simulink model "MX_Gateway"  (Simulink 9.0)
settings: Solver=FixedStepDiscrete  StartTime=0.0  StopTime=100  FixedStep=0.01  RelTol=1e-3
values from neighbouring files (symbols this model uses):
  ACTIVE = Bobcat.Parameter; CreateDefine( ACTIVE                         ,1                          ,'Define' ,'defines.h' )    [defines.m]
  ACTIVE_MODE_ONE = Bobcat.Parameter; CreateDefine( ACTIVE_MODE_ONE                ,19                         ,'Define' ,'defines.h' )    [defines.m]
  AUTHENTICATED = Bobcat.Parameter; CreateDefine( AUTHENTICATED                  ,1                          ,'Define' ,'defines.h' )    [defines.m]
  AUX_EMEA_CONTROLS = Bobcat.Parameter; CreateDefine( AUX_EMEA_CONTROLS  ,1   ,'Define' ,'defines.h' )    [enums.m]
  AUX_FLOW_HIGH = Bobcat.Parameter; CreateDefine( AUX_FLOW_HIGH           ,4  ,'Define' ,'defines.h' )    [enums.m]
  AUX_FLOW_INACTIVE = Bobcat.Parameter; CreateDefine( AUX_FLOW_INACTIVE       ,0  ,'Define' ,'defines.h' )    [enums.m]
  AUX_FLOW_LOW = Bobcat.Parameter; CreateDefine( AUX_FLOW_LOW            ,1  ,'Define' ,'defines.h' )    [enums.m]
  AUX_FLOW_MEDIUM = Bobcat.Parameter; CreateDefine( AUX_FLOW_MEDIUM         ,2  ,'Define' ,'defines.h' )    [enums.m]
  AUX_FLOW_STANDARD = Bobcat.Parameter; CreateDefine( AUX_FLOW_STANDARD       ,3  ,'Define' ,'defines.h' )    [enums.m]
  AUX_NON_FUNCTIONAL = Bobcat.Parameter; CreateDefine( AUX_NON_FUNCTIONAL ,255 ,'Define' ,'defines.h' )    [enums.m]
  BASE_ACTIVE = Bobcat.Parameter; CreateDefine( BASE_ACTIVE                    ,253                        ,'Define' ,'defines.h' )    [defines.m]
  BASE_DETENT = Bobcat.Parameter; CreateDefine( BASE_DETENT                    ,1                          ,'Define' ,'defines.h' )    [defines.m]
  BEEP_NONE = Bobcat.Parameter; CreateDefine( BEEP_NONE                      ,255                        ,'Define' ,'defines.h' )    [defines.m]
  CD_AIR_FILTER_DEBOUNCE_NORMAL = Bobcat.Parameter; CreateAliasCD( CD_AIR_FILTER_DEBOUNCE_NORMAL                        ,uint16(500)                ,'ConstantDataPointer' ,'CONSTANT_DATA' ,'AIR_FILTER_DEBOUNCE_NORMAL')    [cdata.m]    (also in hc12_cdata.m; m4_cdata.m)
  CD_AIR_FILTER_DEBOUNCE_NOT_CONNECTED = Bobcat.Parameter; CreateAliasCD( CD_AIR_FILTER_DEBOUNCE_NOT_CONNECTED                 ,uint16(3000)               ,'ConstantDataPointer' ,'CONSTANT_DATA' ,'AIR_FILTER_DEBOUNCE_NOT_CONNECTED')    [cdata.m]    (also in hc12_cdata.m; m4_cdata.m)
  CD_AIR_FILTER_DEBOUNCE_PLUGGED = Bobcat.Parameter; CreateAliasCD( CD_AIR_FILTER_DEBOUNCE_PLUGGED                       ,uint16(3000)               ,'ConstantDataPointer' ,'CONSTANT_DATA' ,'AIR_FILTER_DEBOUNCE_PLUGGED')    [cdata.m]    (also in hc12_cdata.m; m4_cdata.m)
  CD_AIR_FILTER_FUNCTION = Bobcat.Parameter; CreateAliasCD( CD_AIR_FILTER_FUNCTION                               ,uint8(255)                 ,'ConstantDataPointer' ,'CONSTANT_DATA' ,'AIR_FILTER_FUNCTION')    [cdata.m]    (also in hc12_cdata.m; m4_cdata.m)
  CD_AIR_FILTER_PLUGGED_WEIGHT = Bobcat.Parameter; CreateAliasCD( CD_AIR_FILTER_PLUGGED_WEIGHT                         ,uint8(2)                   ,'ConstantDataPointer' ,'CONSTANT_DATA' ,'AIR_FILTER_PLUGGED_WEIGHT')    [cdata.m]    (also in hc12_cdata.m; m4_cdata.m)
  CD_AIR_FILTER_UNPLUGGED_WEIGHT = Bobcat.Parameter; CreateAliasCD( CD_AIR_FILTER_UNPLUGGED_WEIGHT                       ,uint8(1)                   ,'ConstantDataPointer' ,'CONSTANT_DATA' ,'AIR_FILTER_UNPLUGGED_WEIGHT')    [cdata.m]    (also in hc12_cdata.m; m4_cdata.m)
  CD_AUTOIDLE_ARMBAR_DELAY = Bobcat.Parameter; CreateAliasCD( CD_AUTOIDLE_ARMBAR_DELAY                             ,uint8(2)                   ,'ConstantDataPointer' ,'CONSTANT_DATA' ,'AUTOIDLE_ARMBAR_DELAY')    [cdata.m]    (also in hc12_cdata.m; m4_cdata.m)
  CD_AUTOIDLE_FUNCTION = Bobcat.Parameter; CreateAliasCD( CD_AUTOIDLE_FUNCTION                                 ,uint8(255)                 ,'ConstantDataPointer' ,'CONSTANT_DATA' ,'AUTOIDLE_FUNCTION')    [cdata.m]    (also in hc12_cdata.m; m4_cdata.m)
  CD_AUTOIDLE_IDLE_DELAY = Bobcat.Parameter; CreateAliasCD( CD_AUTOIDLE_IDLE_DELAY                               ,uint8(40)                  ,'ConstantDataPointer' ,'CONSTANT_DATA' ,'AUTOIDLE_IDLE_DELAY')    [cdata.m]    (also in hc12_cdata.m; m4_cdata.m)
  CD_AUTOIDLE_LOW_IDLE_START = Bobcat.Parameter; CreateAliasCD( CD_AUTOIDLE_LOW_IDLE_START                           ,uint8(255)                 ,'ConstantDataPointer' ,'CONSTANT_DATA' ,'AUTOIDLE_LOW_IDLE_START')    [cdata.m]    (also in hc12_cdata.m; m4_cdata.m)
  CD_AUTOIDLE_PRESSURE_OFF = Bobcat.Parameter; CreateAliasCD( CD_AUTOIDLE_PRESSURE_OFF                             ,uint16(300)                ,'ConstantDataPointer' ,'CONSTANT_DATA' ,'AUTOIDLE_PRESSURE_OFF')    [cdata.m]    (also in hc12_cdata.m; m4_cdata.m)
  CD_AUTOIDLE_PRESSURE_ON = Bobcat.Parameter; CreateAliasCD( CD_AUTOIDLE_PRESSURE_ON                              ,uint16(270)                ,'ConstantDataPointer' ,'CONSTANT_DATA' ,'AUTOIDLE_PRESSURE_ON')    [cdata.m]    (also in hc12_cdata.m; m4_cdata.m)
  CD_AUTOSHIFT_FUNCTION = Bobcat.Parameter; CreateAliasCD( CD_AUTOSHIFT_FUNCTION                                ,uint8(255)                 ,'ConstantDataPointer' ,'CONSTANT_DATA' ,'AUTOSHIFT_FUNCTION')    [cdata.m]    (also in hc12_cdata.m; m4_cdata.m)
  CD_AUTOSHIFT_PHIGH1 = Bobcat.Parameter; CreateAliasCD( CD_AUTOSHIFT_PHIGH1                                  ,uint16(205)                ,'ConstantDataPointer' ,'CONSTANT_DATA' ,'AUTOSHIFT_PHIGH1')    [cdata.m]    (also in hc12_cdata.m; m4_cdata.m)
  CD_AUTOSHIFT_PHIGH2 = Bobcat.Parameter; CreateAliasCD( CD_AUTOSHIFT_PHIGH2                                  ,uint16(193)                ,'ConstantDataPointer' ,'CONSTANT_DATA' ,'AUTOSHIFT_PHIGH2')    [cdata.m]    (also in hc12_cdata.m; m4_cdata.m)
  CD_AUTOSHIFT_PHIGH_TEMP1 = Bobcat.Parameter; CreateAliasCD( CD_AUTOSHIFT_PHIGH_TEMP1                             ,int16(70)                  ,'ConstantDataPointer' ,'CONSTANT_DATA' ,'AUTOSHIFT_PHIGH_TEMP1')    [cdata.m]    (also in hc12_cdata.m; m4_cdata.m)
  CD_AUTOSHIFT_PHIGH_TEMP2 = Bobcat.Parameter; CreateAliasCD( CD_AUTOSHIFT_PHIGH_TEMP2                             ,int16(160)                 ,'ConstantDataPointer' ,'CONSTANT_DATA' ,'AUTOSHIFT_PHIGH_TEMP2')    [cdata.m]    (also in hc12_cdata.m; m4_cdata.m)
  CD_AUTOSHIFT_PLOW1 = Bobcat.Parameter; CreateAliasCD( CD_AUTOSHIFT_PLOW1                                   ,uint16(130)                ,'ConstantDataPointer' ,'CONSTANT_DATA' ,'AUTOSHIFT_PLOW1')    [cdata.m]    (also in hc12_cdata.m; m4_cdata.m)
  CD_AUTOSHIFT_PLOW2 = Bobcat.Parameter; CreateAliasCD( CD_AUTOSHIFT_PLOW2                                   ,uint16(100)                ,'ConstantDataPointer' ,'CONSTANT_DATA' ,'AUTOSHIFT_PLOW2')    [cdata.m]    (also in hc12_cdata.m; m4_cdata.m)
  CD_AUTOSHIFT_PLOW_TEMP1 = Bobcat.Parameter; CreateAliasCD( CD_AUTOSHIFT_PLOW_TEMP1                              ,int16(20)                  ,'ConstantDataPointer' ,'CONSTANT_DATA' ,'AUTOSHIFT_PLOW_TEMP1')    [cdata.m]    (also in hc12_cdata.m; m4_cdata.m)
  CD_AUTOSHIFT_PLOW_TEMP2 = Bobcat.Parameter; CreateAliasCD( CD_AUTOSHIFT_PLOW_TEMP2                              ,int16(80)                  ,'ConstantDataPointer' ,'CONSTANT_DATA' ,'AUTOSHIFT_PLOW_TEMP2')    [cdata.m]    (also in hc12_cdata.m; m4_cdata.m)
  CD_AUTOSHIFT_TRAVEL_DELAY = Bobcat.Parameter; CreateAliasCD( CD_AUTOSHIFT_TRAVEL_DELAY                            ,uint8(32)                  ,'ConstantDataPointer' ,'CONSTANT_DATA' ,'AUTOSHIFT_TRAVEL_DELAY')    [cdata.m]    (also in hc12_cdata.m; m4_cdata.m)
  CD_AUX2_CRACKPOINT_CURRENT = Bobcat.Parameter; CreateAliasCD( CD_AUX2_CRACKPOINT_CURRENT                           ,fi(0,0,16,12,'hex','0c28') ,'ConstantDataPointer' ,'CONSTANT_DATA' ,'AUX2_CRACKPOINT_CURRENT')    [cdata.m]    (also in hc12_cdata.m; m4_cdata.m)
  CD_AUX2_FLOW_LOW_FUNCTION = Bobcat.Parameter; CreateAliasCD( CD_AUX2_FLOW_LOW_FUNCTION                            ,uint8(255)                 ,'ConstantDataPointer' ,'CONSTANT_DATA' ,'AUX2_FLOW_LOW_FUNCTION')    [cdata.m]    (also in hc12_cdata.m; m4_cdata.m)
  CD_AUX2_FLOW_MED_FUNCTION = Bobcat.Parameter; CreateAliasCD( CD_AUX2_FLOW_MED_FUNCTION                            ,uint8(255)                 ,'ConstantDataPointer' ,'CONSTANT_DATA' ,'AUX2_FLOW_MED_FUNCTION')    [cdata.m]    (also in hc12_cdata.m; m4_cdata.m)
  CD_AUX2_HI_FLOW_PERCENT = Bobcat.Parameter; CreateAliasCD( CD_AUX2_HI_FLOW_PERCENT                              ,fi(0,0,16,16,'hex','ffff') ,'ConstantDataPointer' ,'CONSTANT_DATA' ,'AUX2_HI_FLOW_PERCENT')    [cdata.m]    (also in hc12_cdata.m; m4_cdata.m)
  CD_AUX2_LOW_FLOW_PERCENT = Bobcat.Parameter; CreateAliasCD( CD_AUX2_LOW_FLOW_PERCENT                             ,fi(0,0,16,16,'hex','7333') ,'ConstantDataPointer' ,'CONSTANT_DATA' ,'AUX2_LOW_FLOW_PERCENT')    [cdata.m]    (also in hc12_cdata.m; m4_cdata.m)
  CD_AUX2_MAX_FLOW_CURRENT = Bobcat.Parameter; CreateAliasCD( CD_AUX2_MAX_FLOW_CURRENT                             ,fi(0,0,16,12,'hex','14cc') ,'ConstantDataPointer' ,'CONSTANT_DATA' ,'AUX2_MAX_FLOW_CURRENT')    [cdata.m]    (also in hc12_cdata.m; m4_cdata.m)
  CD_AUX2_MED_FLOW_PERCENT = Bobcat.Parameter; CreateAliasCD( CD_AUX2_MED_FLOW_PERCENT                             ,fi(0,0,16,16,'hex','9999') ,'ConstantDataPointer' ,'CONSTANT_DATA' ,'AUX2_MED_FLOW_PERCENT')    [cdata.m]    (also in hc12_cdata.m; m4_cdata.m)
  CD_AUX_CRACKPOINT_CURRENT = Bobcat.Parameter; CreateAliasCD( CD_AUX_CRACKPOINT_CURRENT                            ,fi(0,0,16,12,'hex','0000') ,'ConstantDataPointer' ,'CONSTANT_DATA' ,'AUX_CRACKPOINT_CURRENT')    [cdata.m]    (also in hc12_cdata.m; m4_cdata.m)
  CD_AUX_DETENT_FLASH_TIME = Bobcat.Parameter; CreateAliasCD( CD_AUX_DETENT_FLASH_TIME                             ,uint16(72)                 ,'ConstantDataPointer' ,'CONSTANT_DATA' ,'AUX_DETENT_FLASH_TIME')    [cdata.m]    (also in hc12_cdata.m; m4_cdata.m)
  CD_AUX_DETENT_HOLD_TIME = Bobcat.Parameter; CreateAliasCD( CD_AUX_DETENT_HOLD_TIME                              ,uint16(100)                ,'ConstantDataPointer' ,'CONSTANT_DATA' ,'AUX_DETENT_HOLD_TIME')    [cdata.m]    (also in hc12_cdata.m; m4_cdata.m)
  CD_AUX_FULL_ON_AUX1 = Bobcat.Parameter; CreateAliasCD( CD_AUX_FULL_ON_AUX1                                  ,fi(0,0,16,12,'hex','10cd') ,'ConstantDataPointer' ,'CONSTANT_DATA' ,'AUX_FULL_ON_AUX1')    [cdata.m]    (also in hc12_cdata.m; m4_cdata.m)
  CD_AUX_FULL_ON_AUX2 = Bobcat.Parameter; CreateAliasCD( CD_AUX_FULL_ON_AUX2                                  ,fi(0,0,16,12,'hex','139d') ,'ConstantDataPointer' ,'CONSTANT_DATA' ,'AUX_FULL_ON_AUX2')    [cdata.m]    (also in hc12_cdata.m; m4_cdata.m)
  CD_AUX_FULL_ON_AUX3 = Bobcat.Parameter; CreateAliasCD( CD_AUX_FULL_ON_AUX3                                  ,fi(0,0,16,12,'hex','17d7') ,'ConstantDataPointer' ,'CONSTANT_DATA' ,'AUX_FULL_ON_AUX3')    [cdata.m]    (also in hc12_cdata.m; m4_cdata.m)
  CD_AUX_FUNCTION = Bobcat.Parameter; CreateAliasCD( CD_AUX_FUNCTION                                      ,uint8(0)                   ,'ConstantDataPointer' ,'CONSTANT_DATA' ,'AUX_FUNCTION')    [cdata.m]    (also in hc12_cdata.m; m4_cdata.m)
  CD_AUX_RATE_LIMIT_CRACKPOINT = Bobcat.Parameter; CreateAliasCD( CD_AUX_RATE_LIMIT_CRACKPOINT                         ,fi(0,0,16,12,'hex','0800') ,'ConstantDataPointer' ,'CONSTANT_DATA' ,'AUX_RATE_LIMIT_CRACKPOINT')    [cdata.m]    (also in hc12_cdata.m; m4_cdata.m)
  CD_AUX_RATE_LIMIT_CRACKPOINT_RATE = Bobcat.Parameter; CreateAliasCD( CD_AUX_RATE_LIMIT_CRACKPOINT_RATE                    ,fi(0,1,32,12,'int',102400) ,'ConstantDataPointer' ,'CONSTANT_DATA' ,'AUX_RATE_LIMIT_CRACKPOINT_RATE')    [cdata.m]    (also in hc12_cdata.m; m4_cdata.m)
  CD_AUX_RATE_LIMIT_FUNCTION = Bobcat.Parameter; CreateAliasCD( CD_AUX_RATE_LIMIT_FUNCTION                           ,uint8(255)                 ,'ConstantDataPointer' ,'CONSTANT_DATA' ,'AUX_RATE_LIMIT_FUNCTION')    [cdata.m]    (also in hc12_cdata.m; m4_cdata.m)
  CD_AUX_RATE_LIMIT_MAX_ENG_SPD = Bobcat.Parameter; CreateAliasCD( CD_AUX_RATE_LIMIT_MAX_ENG_SPD                        ,uint16(1600)               ,'ConstantDataPointer' ,'CONSTANT_DATA' ,'AUX_RATE_LIMIT_MAX_ENG_SPD')    [cdata.m]    (also in hc12_cdata.m; m4_cdata.m)
  CD_AUX_RATE_LIMIT_MIN_ENG_SPD = Bobcat.Parameter; CreateAliasCD( CD_AUX_RATE_LIMIT_MIN_ENG_SPD                        ,uint16(100)                ,'ConstantDataPointer' ,'CONSTANT_DATA' ,'AUX_RATE_LIMIT_MIN_ENG_SPD')    [cdata.m]    (also in hc12_cdata.m; m4_cdata.m)
  CD_AUX_RATE_LIMIT_NORMAL_RATE = Bobcat.Parameter; CreateAliasCD( CD_AUX_RATE_LIMIT_NORMAL_RATE                        ,fi(0,1,32,12,'int',2048)   ,'ConstantDataPointer' ,'CONSTANT_DATA' ,'AUX_RATE_LIMIT_NORMAL_RATE')    [cdata.m]    (also in hc12_cdata.m; m4_cdata.m)
  CD_AUXPOT_CAL_DEADBAND_HIGH = Bobcat.Parameter; CreateAliasCD( CD_AUXPOT_CAL_DEADBAND_HIGH                          ,uint16(574)                ,'ConstantDataPointer' ,'CONSTANT_DATA' ,'AUXPOT_CAL_DEADBAND_HIGH')    [cdata.m]    (also in hc12_cdata.m; m4_cdata.m)
  CD_AUXPOT_CAL_DEADBAND_LOW = Bobcat.Parameter; CreateAliasCD( CD_AUXPOT_CAL_DEADBAND_LOW                           ,uint16(424)                ,'ConstantDataPointer' ,'CONSTANT_DATA' ,'AUXPOT_CAL_DEADBAND_LOW')    [cdata.m]    (also in hc12_cdata.m; m4_cdata.m)
  CD_AUXPOT_CENTER_RATIO = Bobcat.Parameter; CreateAliasCD( CD_AUXPOT_CENTER_RATIO                               ,uint16(500)                ,'ConstantDataPointer' ,'CONSTANT_DATA' ,'AUXPOT_CENTER_RATIO')    [cdata.m]    (also in hc12_cdata.m; m4_cdata.m)
  CD_AUXPOT_DEADBAND = Bobcat.Parameter; CreateAliasCD( CD_AUXPOT_DEADBAND                                   ,uint8(38)                  ,'ConstantDataPointer' ,'CONSTANT_DATA' ,'AUXPOT_DEADBAND')    [cdata.m]    (also in hc12_cdata.m; m4_cdata.m)
  CD_AUXPOT_FUNCTION = Bobcat.Parameter; CreateAliasCD( CD_AUXPOT_FUNCTION                                   ,uint8(0)                   ,'ConstantDataPointer' ,'CONSTANT_DATA' ,'AUXPOT_FUNCTION')    [cdata.m]    (also in hc12_cdata.m; m4_cdata.m)
  CD_AUXPOT_RATIO_GAIN = Bobcat.Parameter; CreateAliasCD( CD_AUXPOT_RATIO_GAIN                                 ,uint16(1000)               ,'ConstantDataPointer' ,'CONSTANT_DATA' ,'AUXPOT_RATIO_GAIN')    [cdata.m]    (also in hc12_cdata.m; m4_cdata.m)
  CD_CAN_THROTTLE_FUNCTION = Bobcat.Parameter; CreateAliasCD( CD_CAN_THROTTLE_FUNCTION                             ,uint8(255)                 ,'ConstantDataPointer' ,'CONSTANT_DATA' ,'CAN_THROTTLE_FUNCTION')    [cdata.m]    (also in hc12_cdata.m; m4_cdata.m)
  CD_CAN_THROTTLE_REVERSE = Bobcat.Parameter; CreateAliasCD( CD_CAN_THROTTLE_REVERSE                              ,uint8(255)                 ,'ConstantDataPointer' ,'CONSTANT_DATA' ,'CAN_THROTTLE_REVERSE')    [cdata.m]    (also in hc12_cdata.m; m4_cdata.m)
  CD_CONSOLE_DEBOUNCE_ABSENT = Bobcat.Parameter; CreateAliasCD( CD_CONSOLE_DEBOUNCE_ABSENT                           ,uint16(12)                 ,'ConstantDataPointer' ,'CONSTANT_DATA' ,'CONSOLE_DEBOUNCE_ABSENT')    [cdata.m]    (also in hc12_cdata.m; m4_cdata.m)
  CD_CONSOLE_DEBOUNCE_OORH = Bobcat.Parameter; CreateAliasCD( CD_CONSOLE_DEBOUNCE_OORH                             ,uint16(12)                 ,'ConstantDataPointer' ,'CONSTANT_DATA' ,'CONSOLE_DEBOUNCE_OORH')    [cdata.m]    (also in hc12_cdata.m; m4_cdata.m)
  CD_CONSOLE_DEBOUNCE_OORL = Bobcat.Parameter; CreateAliasCD( CD_CONSOLE_DEBOUNCE_OORL                             ,uint16(12)                 ,'ConstantDataPointer' ,'CONSTANT_DATA' ,'CONSOLE_DEBOUNCE_OORL')    [cdata.m]    (also in hc12_cdata.m; m4_cdata.m)
  CD_CONSOLE_DEBOUNCE_PRESENT = Bobcat.Parameter; CreateAliasCD( CD_CONSOLE_DEBOUNCE_PRESENT                          ,uint16(12)                 ,'ConstantDataPointer' ,'CONSTANT_DATA' ,'CONSOLE_DEBOUNCE_PRESENT')    [cdata.m]    (also in hc12_cdata.m; m4_cdata.m)
  CD_CONSOLE_FUNCTION = Bobcat.Parameter; CreateAliasCD( CD_CONSOLE_FUNCTION                                  ,uint8(0)                   ,'ConstantDataPointer' ,'CONSTANT_DATA' ,'CONSOLE_FUNCTION')    [cdata.m]    (also in hc12_cdata.m; m4_cdata.m)
  CD_CONSOLE_OORH = Bobcat.Parameter; CreateAliasCD( CD_CONSOLE_OORH                                      ,uint16(740)                ,'ConstantDataPointer' ,'CONSTANT_DATA' ,'CONSOLE_OORH')    [cdata.m]    (also in hc12_cdata.m; m4_cdata.m)
  CD_CONSOLE_OORL = Bobcat.Parameter; CreateAliasCD( CD_CONSOLE_OORL                                      ,uint16(10)                 ,'ConstantDataPointer' ,'CONSTANT_DATA' ,'CONSOLE_OORL')    [cdata.m]    (also in hc12_cdata.m; m4_cdata.m)
  CD_CONSOLE_PRESENT_HIGH = Bobcat.Parameter; CreateAliasCD( CD_CONSOLE_PRESENT_HIGH                              ,uint16(515)                ,'ConstantDataPointer' ,'CONSTANT_DATA' ,'CONSOLE_PRESENT_HIGH')    [cdata.m]    (also in hc12_cdata.m; m4_cdata.m)
  CD_CONSOLE_PRESENT_LOW = Bobcat.Parameter; CreateAliasCD( CD_CONSOLE_PRESENT_LOW                               ,uint16(375)                ,'ConstantDataPointer' ,'CONSTANT_DATA' ,'CONSOLE_PRESENT_LOW')    [cdata.m]    (also in hc12_cdata.m; m4_cdata.m)
  CD_DELUXE_GEN5_5_FUNCTION = Bobcat.Parameter; CreateAliasCD( CD_DELUXE_GEN5_5_FUNCTION                            ,uint8(255)                 ,'ConstantDataPointer' ,'CONSTANT_DATA' ,'DELUXE_GEN5_5_FUNCTION')    [cdata.m]    (also in hc12_cdata.m; m4_cdata.m)
  CD_DELUXE_GEN5_FUNCTION = Bobcat.Parameter; CreateAliasCD( CD_DELUXE_GEN5_FUNCTION                              ,uint8(255)                 ,'ConstantDataPointer' ,'CONSTANT_DATA' ,'DELUXE_GEN5_FUNCTION')    [cdata.m]    (also in hc12_cdata.m; m4_cdata.m)
  CD_DERATE_ENGINE_SPEED_DEBOUNCE_HIGH = Bobcat.Parameter; CreateAliasCD( CD_DERATE_ENGINE_SPEED_DEBOUNCE_HIGH                 ,uint16(200)                ,'ConstantDataPointer' ,'CONSTANT_DATA' ,'DERATE_ENGINE_SPEED_DEBOUNCE_HIGH')    [cdata.m]    (also in hc12_cdata.m; m4_cdata.m)
  CD_DERATE_ENGINE_SPEED_DEBOUNCE_SHUTDOWN = Bobcat.Parameter; CreateAliasCD( CD_DERATE_ENGINE_SPEED_DEBOUNCE_SHUTDOWN             ,uint16(1000)               ,'ConstantDataPointer' ,'CONSTANT_DATA' ,'DERATE_ENGINE_SPEED_DEBOUNCE_SHUTDOWN')    [cdata.m]    (also in hc12_cdata.m; m4_cdata.m)
  CD_DERATE_ENGINE_SPEED_HIGH_OFFSET = Bobcat.Parameter; CreateAliasCD( CD_DERATE_ENGINE_SPEED_HIGH_OFFSET                   ,uint16(200)                ,'ConstantDataPointer' ,'CONSTANT_DATA' ,'DERATE_ENGINE_SPEED_HIGH_OFFSET')    [cdata.m]    (also in hc12_cdata.m; m4_cdata.m)
  CD_DERATE_METHOD = Bobcat.Parameter; CreateAliasCD( CD_DERATE_METHOD                                     ,uint8(0)                   ,'ConstantDataPointer' ,'CONSTANT_DATA' ,'DERATE_METHOD')    [cdata.m]    (also in hc12_cdata.m; m4_cdata.m)
  CD_DUMP_VALVE_FUNCTION = Bobcat.Parameter; CreateAliasCD( CD_DUMP_VALVE_FUNCTION                               ,uint8(255)                 ,'ConstantDataPointer' ,'CONSTANT_DATA' ,'DUMP_VALVE_FUNCTION')    [cdata.m]    (also in hc12_cdata.m; m4_cdata.m)
  CD_DUMP_VALVE_ON_CURRENT = Bobcat.Parameter; CreateAliasCD( CD_DUMP_VALVE_ON_CURRENT                             ,fi(0,0,16,12,'hex','ffff') ,'ConstantDataPointer' ,'CONSTANT_DATA' ,'DUMP_VALVE_ON_CURRENT')    [cdata.m]    (also in hc12_cdata.m; m4_cdata.m)
  CD_ECO_MODE_THROTTLE_REDUCTION = Bobcat.Parameter; CreateAliasCD( CD_ECO_MODE_THROTTLE_REDUCTION                       ,uint16(850)                ,'ConstantDataPointer' ,'CONSTANT_DATA' ,'ECO_MODE_THROTTLE_REDUCTION')    [cdata.m]    (also in hc12_cdata.m; m4_cdata.m)
  CD_ECT_FILTER_GAIN = Bobcat.Parameter; CreateAliasCD( CD_ECT_FILTER_GAIN                                   ,uint8(6)                   ,'ConstantDataPointer' ,'CONSTANT_DATA' ,'ECT_FILTER_GAIN')    [cdata.m]    (also in hc12_cdata.m; m4_cdata.m)
  CD_ECU_FUNCTION = Bobcat.Parameter; CreateAliasCD( CD_ECU_FUNCTION                                      ,uint8(255)                 ,'ConstantDataPointer' ,'CONSTANT_DATA' ,'ECU_FUNCTION')    [cdata.m]    (also in hc12_cdata.m; m4_cdata.m)
  CD_ECU_HOLD_DEBOUNCE_ERROR_ON = Bobcat.Parameter; CreateAliasCD( CD_ECU_HOLD_DEBOUNCE_ERROR_ON                        ,uint16(20)                 ,'ConstantDataPointer' ,'CONSTANT_DATA' ,'ECU_HOLD_DEBOUNCE_ERROR_ON')    [cdata.m]    (also in hc12_cdata.m; m4_cdata.m)
  CD_EMEA_CONTROLS_HOLD_TIME = Bobcat.Parameter; CreateAliasCD( CD_EMEA_CONTROLS_HOLD_TIME                           ,uint16(30)                 ,'ConstantDataPointer' ,'CONSTANT_DATA' ,'EMEA_CONTROLS_HOLD_TIME')    [cdata.m]    (also in hc12_cdata.m; m4_cdata.m)
  CD_EMEA_CONTROLS_OPTION_A = Bobcat.Parameter; CreateAliasCD( CD_EMEA_CONTROLS_OPTION_A                            ,uint8(255)                 ,'ConstantDataPointer' ,'CONSTANT_DATA' ,'EMEA_CONTROLS_OPTION_A')    [cdata.m]    (also in hc12_cdata.m; m4_cdata.m)
  CD_EMEA_CONTROLS_OPTION_C = Bobcat.Parameter; CreateAliasCD( CD_EMEA_CONTROLS_OPTION_C                            ,uint8(255)                 ,'ConstantDataPointer' ,'CONSTANT_DATA' ,'EMEA_CONTROLS_OPTION_C')    [cdata.m]    (also in hc12_cdata.m; m4_cdata.m)
  CD_EMEA_CONTROLS_REVERSE = Bobcat.Parameter; CreateAliasCD( CD_EMEA_CONTROLS_REVERSE                             ,uint8(255)                 ,'ConstantDataPointer' ,'CONSTANT_DATA' ,'EMEA_CONTROLS_REVERSE')    [cdata.m]    (also in hc12_cdata.m; m4_cdata.m)
  CD_EMEA_ELECTRIC_VERSION = Bobcat.Parameter; CreateAliasCD( CD_EMEA_ELECTRIC_VERSION                             ,uint8(0)                   ,'ConstantDataPointer' ,'CONSTANT_DATA' ,'EMEA_ELECTRIC_VERSION')    [cdata.m]    (also in hc12_cdata.m; m4_cdata.m)
  CD_EMEA_POT_CAL_DEADBAND_HIGH = Bobcat.Parameter; CreateAliasCD( CD_EMEA_POT_CAL_DEADBAND_HIGH                        ,uint16(574)                ,'ConstantDataPointer' ,'CONSTANT_DATA' ,'EMEA_POT_CAL_DEADBAND_HIGH')    [cdata.m]    (also in hc12_cdata.m; m4_cdata.m)
  CD_EMEA_POT_CAL_DEADBAND_LOW = Bobcat.Parameter; CreateAliasCD( CD_EMEA_POT_CAL_DEADBAND_LOW                         ,uint16(424)                ,'ConstantDataPointer' ,'CONSTANT_DATA' ,'EMEA_POT_CAL_DEADBAND_LOW')    [cdata.m]    (also in hc12_cdata.m; m4_cdata.m)
  CD_ENGINE_RPM_FACTOR = Bobcat.Parameter; CreateAliasCD( CD_ENGINE_RPM_FACTOR                                 ,uint8(35)                  ,'ConstantDataPointer' ,'CONSTANT_DATA' ,'ENGINE_RPM_FACTOR')    [cdata.m]    (also in hc12_cdata.m; m4_cdata.m)
  CD_ENGINE_SPEED_FILTER_FAST_VALUE = Bobcat.Parameter; CreateAliasCD( CD_ENGINE_SPEED_FILTER_FAST_VALUE                    ,uint8(1)                   ,'ConstantDataPointer' ,'CONSTANT_DATA' ,'ENGINE_SPEED_FILTER_FAST_VALUE')    [cdata.m]    (also in hc12_cdata.m; m4_cdata.m)
  CD_ENGINE_SPEED_FILTER_SLOW_VALUE = Bobcat.Parameter; CreateAliasCD( CD_ENGINE_SPEED_FILTER_SLOW_VALUE                    ,uint8(4)                   ,'ConstantDataPointer' ,'CONSTANT_DATA' ,'ENGINE_SPEED_FILTER_SLOW_VALUE')    [cdata.m]    (also in hc12_cdata.m; m4_cdata.m)
  CD_ENGINE_SPEED_RUNNING = Bobcat.Parameter; CreateAliasCD( CD_ENGINE_SPEED_RUNNING                              ,uint16(800)                ,'ConstantDataPointer' ,'CONSTANT_DATA' ,'ENGINE_SPEED_RUNNING')    [cdata.m]    (also in hc12_cdata.m; m4_cdata.m)
  CD_ENGINE_START_DELAY_DEBOUNCE_RPM_NO_SIGNAL = Bobcat.Parameter; CreateAliasCD( CD_ENGINE_START_DELAY_DEBOUNCE_RPM_NO_SIGNAL         ,uint16(0)                  ,'ConstantDataPointer' ,'CONSTANT_DATA' ,'ENGINE_START_DELAY_DEBOUNCE_RPM_NO_SIGNAL')    [cdata.m]    (also in hc12_cdata.m; m4_cdata.m)
  CD_ENGINE_START_DELAY_OFF_TIME = Bobcat.Parameter; CreateAliasCD( CD_ENGINE_START_DELAY_OFF_TIME                       ,uint16(0)                  ,'ConstantDataPointer' ,'CONSTANT_DATA' ,'ENGINE_START_DELAY_OFF_TIME')    [cdata.m]    (also in hc12_cdata.m; m4_cdata.m)
  CD_ENGINE_START_DELAY_RPM_NO_SIGNAL = Bobcat.Parameter; CreateAliasCD( CD_ENGINE_START_DELAY_RPM_NO_SIGNAL                  ,uint16(0)                  ,'ConstantDataPointer' ,'CONSTANT_DATA' ,'ENGINE_START_DELAY_RPM_NO_SIGNAL')    [cdata.m]    (also in hc12_cdata.m; m4_cdata.m)
  CD_EOP_DEBOUNCE_EXTREMELY_LOW = Bobcat.Parameter; CreateAliasCD( CD_EOP_DEBOUNCE_EXTREMELY_LOW                        ,uint16(50)                 ,'ConstantDataPointer' ,'CONSTANT_DATA' ,'EOP_DEBOUNCE_EXTREMELY_LOW')    [cdata.m]    (also in hc12_cdata.m; m4_cdata.m)
  CD_EOP_DEBOUNCE_NORMAL = Bobcat.Parameter; CreateAliasCD( CD_EOP_DEBOUNCE_NORMAL                               ,uint16(50)                 ,'ConstantDataPointer' ,'CONSTANT_DATA' ,'EOP_DEBOUNCE_NORMAL')    [cdata.m]    (also in hc12_cdata.m; m4_cdata.m)
  CD_EOP_DEBOUNCE_SHUTDOWN = Bobcat.Parameter; CreateAliasCD( CD_EOP_DEBOUNCE_SHUTDOWN                             ,uint16(100)                ,'ConstantDataPointer' ,'CONSTANT_DATA' ,'EOP_DEBOUNCE_SHUTDOWN')    [cdata.m]    (also in hc12_cdata.m; m4_cdata.m)
  CD_EOP_FILTER_VALUE = Bobcat.Parameter; CreateAliasCD( CD_EOP_FILTER_VALUE                                  ,uint8(3)                   ,'ConstantDataPointer' ,'CONSTANT_DATA' ,'EOP_FILTER_VALUE')    [cdata.m]    (also in hc12_cdata.m; m4_cdata.m)
  CD_EOP_FUNCTION = Bobcat.Parameter; CreateAliasCD( CD_EOP_FUNCTION                                      ,uint8(1)                   ,'ConstantDataPointer' ,'CONSTANT_DATA' ,'EOP_FUNCTION')    [cdata.m]    (also in hc12_cdata.m; m4_cdata.m)
  CD_EOP_SWITCH_GAUGE_VALUE = Bobcat.Parameter; CreateAliasCD( CD_EOP_SWITCH_GAUGE_VALUE                            ,uint16(125)                ,'ConstantDataPointer' ,'CONSTANT_DATA' ,'EOP_SWITCH_GAUGE_VALUE')    [cdata.m]    (also in hc12_cdata.m; m4_cdata.m)
  CD_EOP_SWITCH_LOW = Bobcat.Parameter; CreateAliasCD( CD_EOP_SWITCH_LOW                                    ,uint16(50)                 ,'ConstantDataPointer' ,'CONSTANT_DATA' ,'EOP_SWITCH_LOW')    [cdata.m]    (also in hc12_cdata.m; m4_cdata.m)
  CD_EOP_SWITCH_PRESSURE_VALUE = Bobcat.Parameter; CreateAliasCD( CD_EOP_SWITCH_PRESSURE_VALUE                         ,uint16(40)                 ,'ConstantDataPointer' ,'CONSTANT_DATA' ,'EOP_SWITCH_PRESSURE_VALUE')    [cdata.m]    (also in hc12_cdata.m; m4_cdata.m)
  CD_EOT_DEBOUNCE_EXTREMELY_LOW = Bobcat.Parameter; CreateAliasCD( CD_EOT_DEBOUNCE_EXTREMELY_LOW                        ,uint16(2)                  ,'ConstantDataPointer' ,'CONSTANT_DATA' ,'EOT_DEBOUNCE_EXTREMELY_LOW')    [cdata.m]    (also in hc12_cdata.m; m4_cdata.m)
  CD_EOT_DEBOUNCE_LOW = Bobcat.Parameter; CreateAliasCD( CD_EOT_DEBOUNCE_LOW                                  ,uint16(2)                  ,'ConstantDataPointer' ,'CONSTANT_DATA' ,'EOT_DEBOUNCE_LOW')    [cdata.m]    (also in hc12_cdata.m; m4_cdata.m)
  CD_EOT_DEBOUNCE_NORMAL = Bobcat.Parameter; CreateAliasCD( CD_EOT_DEBOUNCE_NORMAL                               ,uint16(2)                  ,'ConstantDataPointer' ,'CONSTANT_DATA' ,'EOT_DEBOUNCE_NORMAL')    [cdata.m]    (also in hc12_cdata.m; m4_cdata.m)
  CD_EOT_EXTREMELY_LOW = Bobcat.Parameter; CreateAliasCD( CD_EOT_EXTREMELY_LOW                                 ,uint16(81)                 ,'ConstantDataPointer' ,'CONSTANT_DATA' ,'EOT_EXTREMELY_LOW')    [cdata.m]    (also in hc12_cdata.m; m4_cdata.m)
  CD_EOT_FUNCTION = Bobcat.Parameter; CreateAliasCD( CD_EOT_FUNCTION                                      ,uint8(255)                 ,'ConstantDataPointer' ,'CONSTANT_DATA' ,'EOT_FUNCTION')    [cdata.m]    (also in hc12_cdata.m; m4_cdata.m)
  CD_EOT_LOW = Bobcat.Parameter; CreateAliasCD( CD_EOT_LOW                                           ,uint16(90)                 ,'ConstantDataPointer' ,'CONSTANT_DATA' ,'EOT_LOW')    [cdata.m]    (also in hc12_cdata.m; m4_cdata.m)
  CD_EOT_TEMPERATURE_HYSTERESIS = Bobcat.Parameter; CreateAliasCD( CD_EOT_TEMPERATURE_HYSTERESIS                        ,uint16(2)                  ,'ConstantDataPointer' ,'CONSTANT_DATA' ,'EOT_TEMPERATURE_HYSTERESIS')    [cdata.m]    (also in hc12_cdata.m; m4_cdata.m)
  CD_FAST_TRAVEL_FUNCTION = Bobcat.Parameter; CreateAliasCD( CD_FAST_TRAVEL_FUNCTION                              ,uint8(255)                 ,'ConstantDataPointer' ,'CONSTANT_DATA' ,'FAST_TRAVEL_FUNCTION')    [cdata.m]    (also in hc12_cdata.m; m4_cdata.m)
  CD_FAST_TRAVEL_THROTTLE_DELAY = Bobcat.Parameter; CreateAliasCD( CD_FAST_TRAVEL_THROTTLE_DELAY                        ,uint16(10)                 ,'ConstantDataPointer' ,'CONSTANT_DATA' ,'FAST_TRAVEL_THROTTLE_DELAY')    [cdata.m]    (also in hc12_cdata.m; m4_cdata.m)
  CD_FAST_TRAVEL_THROTTLE_LIMIT = Bobcat.Parameter; CreateAliasCD( CD_FAST_TRAVEL_THROTTLE_LIMIT                        ,uint16(1000)               ,'ConstantDataPointer' ,'CONSTANT_DATA' ,'FAST_TRAVEL_THROTTLE_LIMIT')    [cdata.m]    (also in hc12_cdata.m; m4_cdata.m)
  CD_FLYWHEEL_TEETH = Bobcat.Parameter; CreateAliasCD( CD_FLYWHEEL_TEETH                                    ,uint8(83)                  ,'ConstantDataPointer' ,'CONSTANT_DATA' ,'FLYWHEEL_TEETH')    [cdata.m]    (also in hc12_cdata.m; m4_cdata.m)
  CD_FUEL_LEVEL_FILTER_FAST_VALUE = Bobcat.Parameter; CreateAliasCD( CD_FUEL_LEVEL_FILTER_FAST_VALUE                      ,uint8(5)                   ,'ConstantDataPointer' ,'CONSTANT_DATA' ,'FUEL_LEVEL_FILTER_FAST_VALUE')    [cdata.m]    (also in hc12_cdata.m; m4_cdata.m)
  CD_FUEL_LEVEL_FILTER_SLOW_VALUE = Bobcat.Parameter; CreateAliasCD( CD_FUEL_LEVEL_FILTER_SLOW_VALUE                      ,uint8(10)                  ,'ConstantDataPointer' ,'CONSTANT_DATA' ,'FUEL_LEVEL_FILTER_SLOW_VALUE')    [cdata.m]    (also in hc12_cdata.m; m4_cdata.m)
  CD_FUEL_PRIMARY_FEEDBACK_FUNCTION = Bobcat.Parameter; CreateAliasCD( CD_FUEL_PRIMARY_FEEDBACK_FUNCTION                    ,uint8(0)                   ,'ConstantDataPointer' ,'CONSTANT_DATA' ,'FUEL_PRIMARY_FEEDBACK_FUNCTION')    [cdata.m]    (also in hc12_cdata.m; m4_cdata.m)
  ... (+246 more)
undefined symbols (not defined in the file or in its folder): sigbuilder_block
undefined symbols (not defined in the file): CD_FUEL_RESOLUTION_TIMER
libraries: simulink x186, MX_Library x107
(part 14 of 48)

== system MX_Gateway/MX_Gtwy_Control/Inputs/Key ==
  din_RunEnter : Inport
  din_RemoteKey : Inport  Port=2
  Key : Stateflow chart  TreatAsAtomicUnit=on
  state_Key : Outport
  wires:
  Key/1(state_Key) -> state_Key/1  "state_Key"
  din_RunEnter/1 -> Key/1(din_RunEnter)  "din_RunEnter"
  din_RemoteKey/1 -> Key/2(din_RemoteKey)  "din_RemoteKey"

== system MX_Gateway/MX_Gtwy_Control/Inputs/Load_Moment ==
  state_System : Inport
  ain_LoadMoment : Inport  Port=2
  ain_SensorSupply2 : Inport  Port=3
  din_OWDEnableSw : Inport  Port=4
  state_SensorSupply2 : Inport  Port=5
  Compare To Constant : simulink/Logic and Bit Operations/Compare To Constant  relop===  const=SYS_NORMAL  OutDataTypeStr=boolean
  Compare To Constant1 : simulink/Logic and Bit Operations/Compare To Constant  relop===  const=SYS_NORMAL  OutDataTypeStr=boolean
  Compare to Switch : simulink/Logic and Bit Operations/Compare To Constant  relop===  const=SWITCH_INPUT  OutDataTypeStr=boolean  ZeroCross=off
  Compare to Switch1 : simulink/Logic and Bit Operations/Compare To Constant  relop===  const=SENDER  OutDataTypeStr=boolean  ZeroCross=off
  Compare to Switch2 : simulink/Logic and Bit Operations/Compare To Constant  relop===  const=SWITCH_INPUT  OutDataTypeStr=boolean  ZeroCross=off
  Constant1 : Constant  Value=CD_LOAD_MOMENT_FUNCTION  OutDataTypeStr=uint8
  Constant2 : Constant  Value=CD_LOAD_MOMENT_FUNCTION  OutDataTypeStr=uint8
  Constant3 : Constant  Value=CD_LOAD_MOMENT_FUNCTION  OutDataTypeStr=uint8
  Constant4 : Constant  Value=CD_LOAD_MOMENT_FILTER_GAIN  OutDataTypeStr=uint8
  Data Type Conversion : DataTypeConversion  OutDataTypeStr=int16
  LP_Filter_Adj_Gain : MX_Library/LP_Filter_Adj_Gain
  Load_Moment_Sensor : Subsystem  RTWFileName=mod_Inputs
      settings: RTWSystemCode=Nonreusable function  RTWFileNameOpts=User specified
  Load_Moment_Switch : Subsystem  RTWFileName=mod_Inputs
      settings: RTWSystemCode=Nonreusable function  RTWFileNameOpts=User specified
  Logical Operator : Logic  AllPortsSameDT=off  OutDataTypeStr=boolean
  Logical Operator1 : Logic  AllPortsSameDT=off  OutDataTypeStr=boolean
  Rate Transition{1..5,53} (6 alike) : RateTransition  Integrity=off
  Switch : Switch  Criteria=u2 ~= 0
  state_LoadMoment : Outport
  bar_LoadMoment : Outport  Port=2  OutputWhenDisabled=reset  InitialOutput=[INIT_NORMAL]
  volts_LoadMoment : Outport  Port=3  OutputWhenDisabled=reset  InitialOutput=[INIT_NORMAL]
  wires:
  state_System/1 -> Rate Transition1/1, [state_System]
  Rate Transition53/1 -> Load_Moment_Switch/1(ain_LoadMoment)
  Constant2/1 -> Compare to Switch/1
  Compare To Constant/1 -> Logical Operator/1
  Logical Operator/1 -> Load_Moment_Switch/Enable
  ain_LoadMoment/1 -> Rate Transition53/1, Data Type Conversion/1
  Rate Transition1/1 -> Compare To Constant/1
  Compare to Switch/1 -> Logical Operator/2
  Compare To Constant1/1 -> Logical Operator1/1
  Rate Transition2/1 -> Compare To Constant1/1
  Logical Operator1/1 -> Load_Moment_Sensor/Enable
  Constant1/1 -> Compare to Switch1/1
  Compare to Switch1/1 -> Logical Operator1/2
  Switch/1 -> state_LoadMoment/1
  Load_Moment_Switch/1(state_LoadMoment) -> Switch/1
  Load_Moment_Sensor/1(state_LoadMoment) -> Switch/3
  LP_Filter_Adj_Gain/1 -> Rate Transition3/1
  Rate Transition3/1 -> Load_Moment_Sensor/1(ain_LoadMoment)
  Constant3/1 -> Compare to Switch2/1
  Compare to Switch2/1 -> Switch/2
  ain_SensorSupply2/1 -> Rate Transition4/1
  state_SensorSupply2/1 -> Load_Moment_Sensor/3(state_SensorSupply2)
  [state_System] -> Rate Transition2/1
  Rate Transition4/1 -> Load_Moment_Sensor/2(ain_SensorSupply2)
  Load_Moment_Sensor/2(bar_LoadMoment) -> bar_LoadMoment/1
  Load_Moment_Sensor/3(volts_LoadMoment) -> volts_LoadMoment/1
  din_OWDEnableSw/1 -> Rate Transition5/1
  Rate Transition5/1 -> Load_Moment_Sensor/4(din_OWDEnableSw)
  Constant4/1 -> LP_Filter_Adj_Gain/1
  Data Type Conversion/1 -> LP_Filter_Adj_Gain/2

== system MX_Gateway/MX_Gtwy_Control/Inputs/Load_Sense ==
  state_System : Inport
  ain_SensorSupply2 : Inport  Port=2
  ain_SensorSupply1 : Inport  Port=3
  ain_LoadSense : Inport  Port=4
  state_SensorSupply1 : Inport  Port=5
  state_SensorSupply2 : Inport  Port=6
  Compare To Constant : simulink/Logic and Bit Operations/Compare To Constant  relop===  const=SYS_NORMAL  OutDataTypeStr=boolean
  CompareToConstant1 : simulink/Logic and Bit Operations/Compare To Constant  relop=~=  const=NON_FUNCTIONAL  OutDataTypeStr=boolean  ZeroCross=off
  Constant1 : Constant  Value=CD_LOADSENSE_FUNCTION
  Constant2 : Constant  Value=CD_LOADSENSE_FILTER_GAIN
  Data Type Conversion : DataTypeConversion  OutDataTypeStr=int16
  LP_Filter_Adj_Gain : MX_Library/LP_Filter_Adj_Gain
  Load_Sense : Subsystem
  Logical Operator1 : Logic  AllPortsSameDT=off  OutDataTypeStr=boolean
  psi_LoadSense : Outport
  state_LoadSense : Outport  Port=2
  volts_LoadSense : Outport  Port=3
  bar_LoadSense : Outport  Port=4
  wires:
  ain_SensorSupply2/1 -> Load_Sense/1(ain_SensorSupply2)
  Load_Sense/1(psi_LoadSense) -> psi_LoadSense/1
  Load_Sense/3(state_LoadSense) -> state_LoadSense/1
  ain_SensorSupply1/1 -> Load_Sense/3(ain_SensorSupply1)
  Load_Sense/4(volts_LoadSense) -> volts_LoadSense/1
  state_System/1 -> Compare To Constant/1
  CompareToConstant1/1 -> Logical Operator1/1
  Constant1/1 -> CompareToConstant1/1
  Compare To Constant/1 -> Logical Operator1/2
  Logical Operator1/1 -> Load_Sense/Enable
  Load_Sense/2(bar_LoadSense) -> bar_LoadSense/1
  state_SensorSupply1/1 -> Load_Sense/4(state_SensorSupply1)
  state_SensorSupply2/1 -> Load_Sense/5(state_SensorSupply2)
  Constant2/1 -> LP_Filter_Adj_Gain/1
  Data Type Conversion/1 -> LP_Filter_Adj_Gain/2
  LP_Filter_Adj_Gain/1 -> Load_Sense/2(ain_LoadSense)
  ain_LoadSense/1 -> Data Type Conversion/1

== system MX_Gateway/MX_Gtwy_Control/Inputs/Offset_Pot ==
  state_System : Inport
  ain_OffsetPot : Inport  Port=2
  ain_SensorSupply2 : Inport  Port=3
  msgAge_BJM2Left : Inport  Port=4
  state_JoystickEnable : Inport  Port=5
  state_Console : Inport  Port=6
  state_Engine : Inport  Port=7
  state_SensorSupply2 : Inport  Port=8
  cmd_PotLeft : Inport  Port=9
  CAN_Pot : Subsystem
  Data Type Conversion3 : DataTypeConversion  OutDataTypeStr=uint16
  Multiport Switch : MultiPortSwitch  DataPortOrder=Zero-based contiguous  Inputs=2  zeroidx=on
  Multiport Switch1 : MultiPortSwitch  DataPortOrder=Zero-based contiguous  Inputs=2  zeroidx=on
  Multiport Switch2 : MultiPortSwitch  DataPortOrder=Zero-based contiguous  Inputs=2  zeroidx=on
  Normal_Pot : Subsystem
  Pot_Selection : Truth Table  TreatAsAtomicUnit=on
  Saturation : Saturate  UpperLimit=1000  LowerLimit=0
      settings: AttributesFormatString=Upper Limit = %<UpperLimit>\nLower Limit = %<LowerLimit>
  state_OffsetPot : Outport
  position_OffsetPot : Outport  Port=2
  rm_OffsetPot : Outport  Port=3
  wires:
  ain_OffsetPot/1 -> Normal_Pot/1(ain_OffsetPot)
  state_Console/1 -> CAN_Pot/1(state_Console), Normal_Pot/3(state_Console)
  state_Engine/1 -> Normal_Pot/4(state_Engine), CAN_Pot/2(state_Engine)
  Normal_Pot/1(state_OffsetPot) -> Multiport Switch/2
  state_JoystickEnable/1 -> Normal_Pot/5(state_JoystickEnable), CAN_Pot/3(state_JoystickEnable)
  ain_SensorSupply2/1 -> Normal_Pot/2(ain_SensorSupply2)
  Saturation/1 -> position_OffsetPot/1
  state_SensorSupply2/1 -> Normal_Pot/6(state_SensorSupply2)
  state_System/1 -> Pot_Selection/1(state_System)
  Pot_Selection/1(select_Position) -> Multiport Switch2/1, Multiport Switch1/1, Multiport Switch/1
  Multiport Switch/1 -> state_OffsetPot/1
  Multiport Switch1/1 -> Data Type Conversion3/1
  Normal_Pot/2(position_OffsetPot) -> Multiport Switch1/2
  Multiport Switch2/1 -> rm_OffsetPot/1
  CAN_Pot/1(state_OffsetPot) -> Multiport Switch/3
  Normal_Pot/3(rm_OffsetPot) -> Multiport Switch2/2
  CAN_Pot/2(position_OffsetPot) -> Multiport Switch1/3
  CAN_Pot/3(rm_OffsetPot) -> Multiport Switch2/3
  Pot_Selection/2(enable_Normal) -> Normal_Pot/Enable
  Pot_Selection/3(enable_CAN) -> CAN_Pot/Enable
  msgAge_BJM2Left/1 -> CAN_Pot/4(msgAge_BJM2Left)
  cmd_PotLeft/1 -> CAN_Pot/5(cmd_PotLeft)
  Data Type Conversion3/1 -> Saturation/1

== system MX_Gateway/MX_Gtwy_Control/Inputs/SensorSupply1 ==
  ain_SensorSupply1 : Inport  OutDataTypeStr=uint16
  calc_VBattery : Inport  Port=2
  Sensor_Supply1_State : Stateflow chart  TreatAsAtomicUnit=on
  state_SensorSupply1 : Outport  OutDataTypeStr=uint8
  wires:
  Sensor_Supply1_State/1(state_SensorSupply1) -> state_SensorSupply1/1  "state_SensorSupply1"
  ain_SensorSupply1/1 -> Sensor_Supply1_State/1(ain_SensorSupply1)  "ain_SensorSupply1"
  calc_VBattery/1 -> Sensor_Supply1_State/2(calc_VBattery)

== system MX_Gateway/MX_Gtwy_Control/Inputs/SensorSupply2 ==
  ain_SensorSupply2 : Inport  OutDataTypeStr=uint16
  calc_VBattery : Inport  Port=2
  Sensor_Supply2_State : Stateflow chart  TreatAsAtomicUnit=on
  state_SensorSupply2 : Outport  OutDataTypeStr=uint8
  wires:
  ain_SensorSupply2/1 -> Sensor_Supply2_State/1(ain_SensorSupply2)  "ain_SensorSupply2"
  Sensor_Supply2_State/1(state_SensorSupply2) -> state_SensorSupply2/1  "state_SensorSupply2"
  calc_VBattery/1 -> Sensor_Supply2_State/2(calc_VBattery)

== system MX_Gateway/MX_Gtwy_Control/Inputs/Throttle ==
  adin_ThrottlePrimary : Inport  OutDataTypeStr=uint16
  ain_SensorSupply2 : Inport  Port=2  OutDataTypeStr=uint16
  din_DPFKey : Inport  Port=3
  adin_TravelPressureSwitch : Inport  Port=4  OutDataTypeStr=uint16
  state_SensorSupply2 : Inport  Port=5  OutDataTypeStr=uint8
  state_EcoMode : Inport  Port=6
  FastTravelThrottleLimit : Subsystem
  Primary Throttle : Subsystem
  Throttle_Pos : Stateflow chart  TreatAsAtomicUnit=on
  state_ThrottlePrimary : Outport
  percent_Throttle : Outport  Port=2
  pos_ThrottlePrimary : Outport  Port=3
  derate_Throttle : Outport  Port=4
  wires:
  adin_ThrottlePrimary/1 -> Primary Throttle/1(ain_Throttle)  "adin_ThrottlePrimary"
  Throttle_Pos/1(state_ThrottlePrimary) -> state_ThrottlePrimary/1  "state_ThrottlePrimary"
  ain_SensorSupply2/1 -> Primary Throttle/2(ain_SensorSupply2)  "ain_SensorSupply2"
  Primary Throttle/1(pos_Throttle) -> Throttle_Pos/1(pos_ThrottlePrimary_In)  "pos_ThrottlePrimary_In"
  Primary Throttle/2(rm_Throttle) -> Throttle_Pos/2(rm_ThrottlePrimary)  "rm_ThrottlePrimary"
  Throttle_Pos/2(pos_ThrottlePrimary) -> pos_ThrottlePrimary/1  "pos_ThrottlePrimary"
  state_SensorSupply2/1 -> Throttle_Pos/3(state_SensorSupply2)  "state_SensorSupply2"
  Throttle_Pos/3(derate_Throttle) -> derate_Throttle/1  "derate_Throttle"
  din_DPFKey/1 -> Throttle_Pos/4(din_DPFKey)
  state_EcoMode/1 -> Primary Throttle/3(state_EcoMode)
  Throttle_Pos/4(percent_Throttle) -> FastTravelThrottleLimit/1(in_Throttle)  "percent_Throttle"
  adin_TravelPressureSwitch/1 -> FastTravelThrottleLimit/2(adin_TravelPressureSwitch)
  FastTravelThrottleLimit/1(out_Throttle) -> percent_Throttle/1

== system MX_Gateway/MX_Gtwy_Control/Inputs/VBattery ==
  ain_VBattery : Inport  OutDataTypeStr=uint16
  ain_PrecisionReference : Inport  Port=2  OutDataTypeStr=uint16
  state_Engine : Inport  Port=3
  flt_EngineSpeed : Inport  Port=4  OutDataTypeStr=uint16
  Constant1 : Constant  Value=CD_PRECISION_REF_NOMINAL_VALUE  OutDataTypeStr=uint16  SampleTime=srate100
  Constant2 : Constant  Value=CD_VBATTERY_FILTER_GAIN  OutDataTypeStr=uint8  SampleTime=srate100
  Constant3 : Constant  Value=CD_VBATTERY_FILTER_DISPLAY_GAIN  OutDataTypeStr=uint8  SampleTime=srate100
  Data Type Conversion1 : DataTypeConversion
  Data Type Conversion2 : DataTypeConversion
  Data Type Conversion3 : DataTypeConversion
  Divide : Product  Inputs=*/  OutDataTypeStr=fixdt(0,16,10)  RndMeth=Nearest
  Divide1 : Product  Inputs=*/  OutDataTypeStr=fixdt(0,16,8)  RndMeth=Nearest
  LP_Filter_Adj_Gain : Subsystem
  LP_Filter_Adj_Gain1 : Subsystem
  VBattery_State : Stateflow chart  TreatAsAtomicUnit=on
  Vbatt : Gain  Gain=CD_VBATTERY_GAIN  ParamDataTypeStr=fixdt(0,16,12)  OutDataTypeStr=fixdt(0,16,8)  RndMeth=Nearest  SampleTime=srate100  SaturateOnIntegerOverflow=on
  state_VBattery : Outport  OutDataTypeStr=uint8
  calc_VBattery : Outport  Port=2
  calcFlt_VBattery : Outport  Port=3  OutDataTypeStr=fixdt(0,16,8)
  state_Alternator : Outport  Port=4  OutDataTypeStr=uint8
  wires:
  ain_VBattery/1 -> Vbatt/1  "ain_VBattery"
  state_Engine/1 -> VBattery_State/2(state_Engine)  "state_Engine"
  Data Type Conversion1/1 -> calcFlt_VBattery/1  "calcFlt_VBattery"
  LP_Filter_Adj_Gain1/1(Output) -> Data Type Conversion1/1
  ain_PrecisionReference/1 -> Divide/1  "ain_PrecisionReference"
  Constant1/1 -> Divide/2
  Divide/1 -> Divide1/2  "adj_PrecisionReference"
  Vbatt/1 -> Data Type Conversion3/1  "calc_VBattery"
  Divide1/1 -> VBattery_State/1(calc_VBattery), calc_VBattery/1, Data Type Conversion2/1  "calc_VBattery"
  LP_Filter_Adj_Gain/1(Output) -> Divide1/1
  flt_EngineSpeed/1 -> VBattery_State/3(flt_EngineSpeed)  "flt_EngineSpeed"
  VBattery_State/1(state_VBattery) -> state_VBattery/1  "state_VBattery"
  VBattery_State/2(state_Alternator) -> state_Alternator/1  "state_Alternator"
  Constant2/1 -> LP_Filter_Adj_Gain/1(Gain)
  Constant3/1 -> LP_Filter_Adj_Gain1/1(Gain)
  Data Type Conversion2/1 -> LP_Filter_Adj_Gain1/2(Input)
  Data Type Conversion3/1 -> LP_Filter_Adj_Gain/2(Input)

== system MX_Gateway/MX_Gtwy_Control/Outputs/Aux_Hydraulics ==
  state_Engine : Inport
  state_AuxEnable : Inport  Port=2
  state_Detent : Inport  Port=3
  aux_Flow : Inport  Port=4
  cmd_AuxRelease : Inport  Port=5
  mode_RightControl : Inport  Port=6
  state_Vbattery : Inport  Port=7
  calc_Vbattery : Inport  Port=8
  position_AuxPot : Inport  Port=9
  state_AuxPot : Inport  Port=10
  position_RightPot : Inport  Port=11
  state_RightPot : Inport  Port=12
  flt_EngineSpeed : Inport  Port=13
  state_System : Inport  Port=14
  afbk_AuxBase : Inport  Port=15
  ifbk_AuxBase : Inport  Port=16
  afbk_AuxRod : Inport  Port=17
  ifbk_AuxRod : Inport  Port=18
  ain_PrecRef : Inport  Port=19
  can_ACD_AuxCommand : Inport  Port=20
  can_AuxEnable_ACD : Inport  Port=21
  flow_Aux1Percent : Inport  Port=22
  Aux_Hydraulics : Subsystem
  CompareToConstant1 : simulink/Logic and Bit Operations/Compare To Constant  relop===  const=SYS_NORMAL  OutDataTypeStr=boolean  ZeroCross=off
  CompareToConstant2 : simulink/Logic and Bit Operations/Compare To Constant  relop=~=  const=NON_FUNCTIONAL  OutDataTypeStr=boolean  ZeroCross=off
  Constant1 : Constant  Value=CD_AUX_FUNCTION
  Logical Operator : Logic  AllPortsSameDT=off  OutDataTypeStr=boolean
  hs_AuxBase : Outport  OutputWhenDisabled=reset  InitialOutput=[0]
  ls_AuxBase : Outport  Port=2  OutputWhenDisabled=reset  InitialOutput=[0]
  errorCC_AuxBase : Outport  Port=3  OutputWhenDisabled=reset  InitialOutput=[0]
  calc_ifbk_AuxBase : Outport  Port=4  OutputWhenDisabled=reset  InitialOutput=[0]
  state_AuxBase : Outport  Port=5  OutputWhenDisabled=reset  InitialOutput=[0]
  hs_AuxRod : Outport  Port=6  OutputWhenDisabled=reset  InitialOutput=[0]
  ls_AuxRod : Outport  Port=7  OutputWhenDisabled=reset  InitialOutput=[INIT_NORMAL]
  errorCC_AuxRod : Outport  Port=8  OutputWhenDisabled=reset  InitialOutput=[0]
  calc_ifbk_AuxRod : Outport  Port=9  OutputWhenDisabled=reset  InitialOutput=[INIT_NORMAL]
  state_AuxRod : Outport  Port=10
  state_AuxFlow : Outport  Port=11  OutputWhenDisabled=reset  InitialOutput=[0]
  wires:
  state_System/1 -> CompareToConstant1/1
  CompareToConstant1/1 -> Logical Operator/2
  state_Engine/1 -> Aux_Hydraulics/1(state_Engine)
  Aux_Hydraulics/1(hs_AuxBase) -> hs_AuxBase/1
  Aux_Hydraulics/2(ls_AuxBase) -> ls_AuxBase/1
  Aux_Hydraulics/3(errorCC_AuxBase) -> errorCC_AuxBase/1
  Aux_Hydraulics/4(calc_ifbk_AuxBase) -> calc_ifbk_AuxBase/1
  Aux_Hydraulics/5(state_AuxBase) -> state_AuxBase/1
  Aux_Hydraulics/6(hs_AuxRod) -> hs_AuxRod/1
  Aux_Hydraulics/7(ls_AuxRod) -> ls_AuxRod/1
  Aux_Hydraulics/8(errorCC_AuxRod) -> errorCC_AuxRod/1
  Aux_Hydraulics/9(calc_ifbk_AuxRod) -> calc_ifbk_AuxRod/1
  Aux_Hydraulics/10(state_AuxRod) -> state_AuxRod/1
  Logical Operator/1 -> Aux_Hydraulics/Enable
  state_AuxEnable/1 -> Aux_Hydraulics/2(state_AuxEnable)
  aux_Flow/1 -> Aux_Hydraulics/3(aux_Flow)
  state_Vbattery/1 -> Aux_Hydraulics/4(state_Vbattery)
  calc_Vbattery/1 -> Aux_Hydraulics/5(calc_Vbattery)
  position_AuxPot/1 -> Aux_Hydraulics/6(position_AuxPot)
  state_Detent/1 -> Aux_Hydraulics/7(state_Detent)
  state_AuxPot/1 -> Aux_Hydraulics/8(state_AuxPot)
  afbk_AuxBase/1 -> Aux_Hydraulics/9(afbk_AuxBase)
  ifbk_AuxBase/1 -> Aux_Hydraulics/10(ifbk_AuxBase)
  afbk_AuxRod/1 -> Aux_Hydraulics/11(afbk_AuxRod)
  ifbk_AuxRod/1 -> Aux_Hydraulics/12(ifbk_AuxRod)
  Constant1/1 -> CompareToConstant2/1
  CompareToConstant2/1 -> Logical Operator/1
  ain_PrecRef/1 -> Aux_Hydraulics/13(ain_PrecRef)
  can_ACD_AuxCommand/1 -> Aux_Hydraulics/14(can_ACD_AuxCommand)
  can_AuxEnable_ACD/1 -> Aux_Hydraulics/15(can_AuxEnable_ACD)
  cmd_AuxRelease/1 -> Aux_Hydraulics/16(cmd_AuxRelease)
  Aux_Hydraulics/11(state_AuxFlow) -> state_AuxFlow/1
  flt_EngineSpeed/1 -> Aux_Hydraulics/17(flt_EngineSpeed)
  state_RightPot/1 -> Aux_Hydraulics/19(state_RightPot)
  position_RightPot/1 -> Aux_Hydraulics/18(position_RightPot)
  mode_RightControl/1 -> Aux_Hydraulics/20(mode_RightControl)
  flow_Aux1Percent/1 -> Aux_Hydraulics/21(flow_Aux1Percent)

== system MX_Gateway/MX_Gtwy_Control/Outputs/Buzzer ==
  state_System : Inport  OutDataTypeStr=uint8
  delay_DepthBeep : Inport  Port=2
  state_DepthBeep : Inport  Port=3  OutDataTypeStr=uint8
  Buzzer : Stateflow chart  RTWFileName=mod_Diagnostics
      settings: RTWSystemCode=Nonreusable function  RTWFileNameOpts=User specified
  dout_Buzzer : Outport
  wires:
  Buzzer/1(dout_Buzzer) -> dout_Buzzer/1
  state_System/1 -> Buzzer/1(state_System)
  delay_DepthBeep/1 -> Buzzer/2(delay_DepthBeep)
  state_DepthBeep/1 -> Buzzer/3(state_DepthBeep)
